simulink model "x64_32s_001_4ks"  (Simulink 7.4)
settings: Solver=ode45  StartTime=0.0  StopTime=100000  RelTol=1e-3
undefined symbols (not defined in the file): gcbh, ROACH, x64_32s_001_4ks_beam_mux_order_in_user_data_out, x64_32s_001_4ks_beam_mux_order_out_user_data_out, x64_32s_001_4ks_beam_mux_order_we_user_data_out, x64_32s_001_4ks_first_chan_user_data_out, x64_32s_001_4ks_gbe_dest_ip_user_data_out, x64_32s_001_4ks_gbe_dest_port_user_data_out, x64_32s_001_4ks_gbe_packet_cnt1_user_data_in, x64_32s_001_4ks_gbe_packet_cnt_user_data_in, x64_32s_001_4ks_heap_size_user_data_out, x64_32s_001_4ks_inst_seng_id0_user_data_in, x64_32s_001_4ks_last_chan_user_data_out, x64_32s_001_4ks_n_beams_user_data_out, x64_32s_001_4ks_qdr_in_cnt_user_data_in, x64_32s_001_4ks_status_user_data_in, x64_32s_001_4ks_x_window_mult_0_coeff_user_data_out, x64_32s_001_4ks_x_window_mult_1_coeff_user_data_out, x64_32s_001_4ks_x_window_mult_2_coeff_user_data_out, x64_32s_001_4ks_x_window_mult_3_coeff_user_data_out, x64_32s_001_4ks_xaui_cnt0_user_data_in, x64_32s_001_4ks_xaui_error_cnt0_user_data_in, x64_32s_001_4ks_xauibuf_out_cnt_user_data_in, x64_32s_001_4ks_xauibuf_window_cnt_user_data_in, x64_32s_001_4ks_y_window_mult_0_coeff_user_data_out, x64_32s_001_4ks_y_window_mult_1_coeff_user_data_out, x64_32s_001_4ks_y_window_mult_2_coeff_user_data_out, x64_32s_001_4ks_y_window_mult_3_coeff_user_data_out, x64_32s_001_4ks_y_window_mult_4_coeff_user_data_out, x64_32s_001_4ks_y_window_mult_5_coeff_user_data_out, x64_32s_001_4ks_y_window_mult_6_coeff_user_data_out, x64_32s_001_4ks_y_window_mult_7_coeff_user_data_out, xlBlockLoadCallback
libraries: xbsIndex_r4 x8559, casper_library_misc x308, casper_library_delays x33, casper_library_reorder x16, xps_library x5, ox_lib x1
(part 14 of 100)

== system x64_32s_001_4ks/beam_tvg/pow_sim_mux10 ==
  sync : Inport  OutDataType=fixdt(1, 16)  OutScaling=2^0
  din : Inport  Port=2  OutDataType=fixdt(1, 16)  OutScaling=2^0
  tvg_en : Inport  Port=3  OutDataType=fixdt(1, 16)  OutScaling=2^0
  new_window : Inport  Port=4  OutDataType=fixdt(1, 16)  OutScaling=2^0
  Convert : xbsIndex_r4/Convert  arith_type=Unsigned  n_bits=8  bin_pt=0  quantization=Truncate  overflow=Wrap  latency=0  dbl_ovrd=off  pipeline=off  block_type=convert
      settings: block_version=11.4
  Mux : xbsIndex_r4/Mux  inputs=2  en=off  latency=1  precision=Full  arith_type=Unsigned  n_bits=16  bin_pt=14  quantization=Truncate  overflow=Wrap  dbl_ovrd=off  block_type=mux
      settings: block_version=11.4
  beam_ctr : xbsIndex_r4/Counter  cnt_type=Free Running  cnt_to=Inf  operation=Up  start_count=0  cnt_by_val=1  arith_type=Unsigned  n_bits=ant_bits  bin_pt=0  load_pin=off  rst=on  en=off  explicit_period=on  period=1  dbl_ovrd=off  use_behavioral_HDL=off  implementation=Fabric  block_type=counter
      settings: block_version=11.4
  chan_ctr : xbsIndex_r4/Counter  cnt_type=Free Running  cnt_to=Inf  operation=Up  start_count=0  cnt_by_val=1  arith_type=Unsigned  n_bits=16  bin_pt=0  load_pin=off  rst=on  en=on  explicit_period=on  period=1  dbl_ovrd=off  use_behavioral_HDL=off  implementation=Fabric  block_type=counter
      settings: block_version=11.4
  imag : xbsIndex_r4/Concat  num_inputs=2  dbl_ovrd=off  block_type=concat
  real : xbsIndex_r4/Concat  num_inputs=3  dbl_ovrd=off  block_type=concat
  tv : xbsIndex_r4/Concat  num_inputs=2  dbl_ovrd=off  block_type=concat
  y_beam_val : xbsIndex_r4/Constant  arith_type=Unsigned  const=y_beam_val  n_bits=8  bin_pt=0  explicit_period=on  period=1  equ=P=C  opselect=C  inp2=PCIN>>17  opr=+  inp1=P  carry=CIN  dbl_ovrd=off  block_type=constant
      settings: block_version=11.4
  zero_pad : xbsIndex_r4/Constant  arith_type=Unsigned  const=0  n_bits=2  bin_pt=0  explicit_period=on  period=1  equ=P=C  opselect=C  inp2=PCIN>>17  opr=+  inp1=P  carry=CIN  dbl_ovrd=off  block_type=constant
      settings: block_version=11.4
  zero_pad1 : xbsIndex_r4/Constant  arith_type=Unsigned  const=0  n_bits=2  bin_pt=0  explicit_period=on  period=1  equ=P=C  opselect=C  inp2=PCIN>>17  opr=+  inp1=P  carry=CIN  dbl_ovrd=off  block_type=constant
      settings: block_version=11.4
  dout : Outport
  wires:
  imag/1 -> tv/2
  zero_pad1/1 -> imag/2
  tv/1 -> Mux/3
  zero_pad/1 -> real/3
  Convert/1 -> real/2
  y_beam_val/1 -> real/1
  din/1 -> Mux/2
  sync/1 -> chan_ctr/1
  beam_ctr/1 -> Convert/1
  tvg_en/1 -> Mux/1
  Mux/1 -> dout/1
  chan_ctr/1 -> imag/1
  real/1 -> tv/1
  new_window/1 -> chan_ctr/2, beam_ctr/1

== system x64_32s_001_4ks/beam_tvg/pow_sim_mux11 ==
  sync : Inport  OutDataType=fixdt(1, 16)  OutScaling=2^0
  din : Inport  Port=2  OutDataType=fixdt(1, 16)  OutScaling=2^0
  tvg_en : Inport  Port=3  OutDataType=fixdt(1, 16)  OutScaling=2^0
  new_window : Inport  Port=4  OutDataType=fixdt(1, 16)  OutScaling=2^0
  Convert : xbsIndex_r4/Convert  arith_type=Unsigned  n_bits=8  bin_pt=0  quantization=Truncate  overflow=Wrap  latency=0  dbl_ovrd=off  pipeline=off  block_type=convert
      settings: block_version=11.4
  Mux : xbsIndex_r4/Mux  inputs=2  en=off  latency=1  precision=Full  arith_type=Unsigned  n_bits=16  bin_pt=14  quantization=Truncate  overflow=Wrap  dbl_ovrd=off  block_type=mux
      settings: block_version=11.4
  beam_ctr : xbsIndex_r4/Counter  cnt_type=Free Running  cnt_to=Inf  operation=Up  start_count=0  cnt_by_val=1  arith_type=Unsigned  n_bits=ant_bits  bin_pt=0  load_pin=off  rst=on  en=off  explicit_period=on  period=1  dbl_ovrd=off  use_behavioral_HDL=off  implementation=Fabric  block_type=counter
      settings: block_version=11.4
  chan_ctr : xbsIndex_r4/Counter  cnt_type=Free Running  cnt_to=Inf  operation=Up  start_count=0  cnt_by_val=1  arith_type=Unsigned  n_bits=16  bin_pt=0  load_pin=off  rst=on  en=on  explicit_period=on  period=1  dbl_ovrd=off  use_behavioral_HDL=off  implementation=Fabric  block_type=counter
      settings: block_version=11.4
  imag : xbsIndex_r4/Concat  num_inputs=2  dbl_ovrd=off  block_type=concat
  real : xbsIndex_r4/Concat  num_inputs=3  dbl_ovrd=off  block_type=concat
  tv : xbsIndex_r4/Concat  num_inputs=2  dbl_ovrd=off  block_type=concat
  y_beam_val : xbsIndex_r4/Constant  arith_type=Unsigned  const=y_beam_val  n_bits=8  bin_pt=0  explicit_period=on  period=1  equ=P=C  opselect=C  inp2=PCIN>>17  opr=+  inp1=P  carry=CIN  dbl_ovrd=off  block_type=constant
      settings: block_version=11.4
  zero_pad : xbsIndex_r4/Constant  arith_type=Unsigned  const=0  n_bits=2  bin_pt=0  explicit_period=on  period=1  equ=P=C  opselect=C  inp2=PCIN>>17  opr=+  inp1=P  carry=CIN  dbl_ovrd=off  block_type=constant
      settings: block_version=11.4
  zero_pad1 : xbsIndex_r4/Constant  arith_type=Unsigned  const=0  n_bits=2  bin_pt=0  explicit_period=on  period=1  equ=P=C  opselect=C  inp2=PCIN>>17  opr=+  inp1=P  carry=CIN  dbl_ovrd=off  block_type=constant
      settings: block_version=11.4
  dout : Outport
  wires:
  imag/1 -> tv/2
  zero_pad1/1 -> imag/2
  tv/1 -> Mux/3
  zero_pad/1 -> real/3
  Convert/1 -> real/2
  y_beam_val/1 -> real/1
  din/1 -> Mux/2
  sync/1 -> chan_ctr/1
  beam_ctr/1 -> Convert/1
  tvg_en/1 -> Mux/1
  Mux/1 -> dout/1
  chan_ctr/1 -> imag/1
  real/1 -> tv/1
  new_window/1 -> chan_ctr/2, beam_ctr/1

== system x64_32s_001_4ks/beam_tvg/pow_sim_mux12 ==
  sync : Inport  OutDataType=fixdt(1, 16)  OutScaling=2^0
  din : Inport  Port=2  OutDataType=fixdt(1, 16)  OutScaling=2^0
  tvg_en : Inport  Port=3  OutDataType=fixdt(1, 16)  OutScaling=2^0
  new_window : Inport  Port=4  OutDataType=fixdt(1, 16)  OutScaling=2^0
  Convert : xbsIndex_r4/Convert  arith_type=Unsigned  n_bits=8  bin_pt=0  quantization=Truncate  overflow=Wrap  latency=0  dbl_ovrd=off  pipeline=off  block_type=convert
      settings: block_version=11.4
  Mux : xbsIndex_r4/Mux  inputs=2  en=off  latency=1  precision=Full  arith_type=Unsigned  n_bits=16  bin_pt=14  quantization=Truncate  overflow=Wrap  dbl_ovrd=off  block_type=mux
      settings: block_version=11.4
  beam_ctr : xbsIndex_r4/Counter  cnt_type=Free Running  cnt_to=Inf  operation=Up  start_count=0  cnt_by_val=1  arith_type=Unsigned  n_bits=ant_bits  bin_pt=0  load_pin=off  rst=on  en=off  explicit_period=on  period=1  dbl_ovrd=off  use_behavioral_HDL=off  implementation=Fabric  block_type=counter
      settings: block_version=11.4
  chan_ctr : xbsIndex_r4/Counter  cnt_type=Free Running  cnt_to=Inf  operation=Up  start_count=0  cnt_by_val=1  arith_type=Unsigned  n_bits=16  bin_pt=0  load_pin=off  rst=on  en=on  explicit_period=on  period=1  dbl_ovrd=off  use_behavioral_HDL=off  implementation=Fabric  block_type=counter
      settings: block_version=11.4
  imag : xbsIndex_r4/Concat  num_inputs=2  dbl_ovrd=off  block_type=concat
  real : xbsIndex_r4/Concat  num_inputs=3  dbl_ovrd=off  block_type=concat
  tv : xbsIndex_r4/Concat  num_inputs=2  dbl_ovrd=off  block_type=concat
  y_beam_val : xbsIndex_r4/Constant  arith_type=Unsigned  const=y_beam_val  n_bits=8  bin_pt=0  explicit_period=on  period=1  equ=P=C  opselect=C  inp2=PCIN>>17  opr=+  inp1=P  carry=CIN  dbl_ovrd=off  block_type=constant
      settings: block_version=11.4
  zero_pad : xbsIndex_r4/Constant  arith_type=Unsigned  const=0  n_bits=2  bin_pt=0  explicit_period=on  period=1  equ=P=C  opselect=C  inp2=PCIN>>17  opr=+  inp1=P  carry=CIN  dbl_ovrd=off  block_type=constant
      settings: block_version=11.4
  zero_pad1 : xbsIndex_r4/Constant  arith_type=Unsigned  const=0  n_bits=2  bin_pt=0  explicit_period=on  period=1  equ=P=C  opselect=C  inp2=PCIN>>17  opr=+  inp1=P  carry=CIN  dbl_ovrd=off  block_type=constant
      settings: block_version=11.4
  dout : Outport
  wires:
  new_window/1 -> beam_ctr/1, chan_ctr/2
  real/1 -> tv/1
  chan_ctr/1 -> imag/1
  Mux/1 -> dout/1
  tvg_en/1 -> Mux/1
  beam_ctr/1 -> Convert/1
  sync/1 -> chan_ctr/1
  din/1 -> Mux/2
  y_beam_val/1 -> real/1
  Convert/1 -> real/2
  zero_pad/1 -> real/3
  tv/1 -> Mux/3
  zero_pad1/1 -> imag/2
  imag/1 -> tv/2

== system x64_32s_001_4ks/beam_tvg/pow_sim_mux13 ==
  sync : Inport  OutDataType=fixdt(1, 16)  OutScaling=2^0
  din : Inport  Port=2  OutDataType=fixdt(1, 16)  OutScaling=2^0
  tvg_en : Inport  Port=3  OutDataType=fixdt(1, 16)  OutScaling=2^0
  new_window : Inport  Port=4  OutDataType=fixdt(1, 16)  OutScaling=2^0
  Convert : xbsIndex_r4/Convert  arith_type=Unsigned  n_bits=8  bin_pt=0  quantization=Truncate  overflow=Wrap  latency=0  dbl_ovrd=off  pipeline=off  block_type=convert
      settings: block_version=11.4
  Mux : xbsIndex_r4/Mux  inputs=2  en=off  latency=1  precision=Full  arith_type=Unsigned  n_bits=16  bin_pt=14  quantization=Truncate  overflow=Wrap  dbl_ovrd=off  block_type=mux
      settings: block_version=11.4
  beam_ctr : xbsIndex_r4/Counter  cnt_type=Free Running  cnt_to=Inf  operation=Up  start_count=0  cnt_by_val=1  arith_type=Unsigned  n_bits=ant_bits  bin_pt=0  load_pin=off  rst=on  en=off  explicit_period=on  period=1  dbl_ovrd=off  use_behavioral_HDL=off  implementation=Fabric  block_type=counter
      settings: block_version=11.4
  chan_ctr : xbsIndex_r4/Counter  cnt_type=Free Running  cnt_to=Inf  operation=Up  start_count=0  cnt_by_val=1  arith_type=Unsigned  n_bits=16  bin_pt=0  load_pin=off  rst=on  en=on  explicit_period=on  period=1  dbl_ovrd=off  use_behavioral_HDL=off  implementation=Fabric  block_type=counter
      settings: block_version=11.4
  imag : xbsIndex_r4/Concat  num_inputs=2  dbl_ovrd=off  block_type=concat
  real : xbsIndex_r4/Concat  num_inputs=3  dbl_ovrd=off  block_type=concat
  tv : xbsIndex_r4/Concat  num_inputs=2  dbl_ovrd=off  block_type=concat
  y_beam_val : xbsIndex_r4/Constant  arith_type=Unsigned  const=y_beam_val  n_bits=8  bin_pt=0  explicit_period=on  period=1  equ=P=C  opselect=C  inp2=PCIN>>17  opr=+  inp1=P  carry=CIN  dbl_ovrd=off  block_type=constant
      settings: block_version=11.4
  zero_pad : xbsIndex_r4/Constant  arith_type=Unsigned  const=0  n_bits=2  bin_pt=0  explicit_period=on  period=1  equ=P=C  opselect=C  inp2=PCIN>>17  opr=+  inp1=P  carry=CIN  dbl_ovrd=off  block_type=constant
      settings: block_version=11.4
  zero_pad1 : xbsIndex_r4/Constant  arith_type=Unsigned  const=0  n_bits=2  bin_pt=0  explicit_period=on  period=1  equ=P=C  opselect=C  inp2=PCIN>>17  opr=+  inp1=P  carry=CIN  dbl_ovrd=off  block_type=constant
      settings: block_version=11.4
  dout : Outport
  wires:
  new_window/1 -> beam_ctr/1, chan_ctr/2
  real/1 -> tv/1
  chan_ctr/1 -> imag/1
  Mux/1 -> dout/1
  tvg_en/1 -> Mux/1
  beam_ctr/1 -> Convert/1
  sync/1 -> chan_ctr/1
  din/1 -> Mux/2
  y_beam_val/1 -> real/1
  Convert/1 -> real/2
  zero_pad/1 -> real/3
  tv/1 -> Mux/3
  zero_pad1/1 -> imag/2
  imag/1 -> tv/2

== system x64_32s_001_4ks/beam_tvg/pow_sim_mux14 ==
  sync : Inport  OutDataType=fixdt(1, 16)  OutScaling=2^0
  din : Inport  Port=2  OutDataType=fixdt(1, 16)  OutScaling=2^0
  tvg_en : Inport  Port=3  OutDataType=fixdt(1, 16)  OutScaling=2^0
  new_window : Inport  Port=4  OutDataType=fixdt(1, 16)  OutScaling=2^0
  Convert : xbsIndex_r4/Convert  arith_type=Unsigned  n_bits=8  bin_pt=0  quantization=Truncate  overflow=Wrap  latency=0  dbl_ovrd=off  pipeline=off  block_type=convert
      settings: block_version=11.4
  Mux : xbsIndex_r4/Mux  inputs=2  en=off  latency=1  precision=Full  arith_type=Unsigned  n_bits=16  bin_pt=14  quantization=Truncate  overflow=Wrap  dbl_ovrd=off  block_type=mux
      settings: block_version=11.4
  beam_ctr : xbsIndex_r4/Counter  cnt_type=Free Running  cnt_to=Inf  operation=Up  start_count=0  cnt_by_val=1  arith_type=Unsigned  n_bits=ant_bits  bin_pt=0  load_pin=off  rst=on  en=off  explicit_period=on  period=1  dbl_ovrd=off  use_behavioral_HDL=off  implementation=Fabric  block_type=counter
      settings: block_version=11.4
  chan_ctr : xbsIndex_r4/Counter  cnt_type=Free Running  cnt_to=Inf  operation=Up  start_count=0  cnt_by_val=1  arith_type=Unsigned  n_bits=16  bin_pt=0  load_pin=off  rst=on  en=on  explicit_period=on  period=1  dbl_ovrd=off  use_behavioral_HDL=off  implementation=Fabric  block_type=counter
      settings: block_version=11.4
  imag : xbsIndex_r4/Concat  num_inputs=2  dbl_ovrd=off  block_type=concat
  real : xbsIndex_r4/Concat  num_inputs=3  dbl_ovrd=off  block_type=concat
  tv : xbsIndex_r4/Concat  num_inputs=2  dbl_ovrd=off  block_type=concat
  y_beam_val : xbsIndex_r4/Constant  arith_type=Unsigned  const=y_beam_val  n_bits=8  bin_pt=0  explicit_period=on  period=1  equ=P=C  opselect=C  inp2=PCIN>>17  opr=+  inp1=P  carry=CIN  dbl_ovrd=off  block_type=constant
      settings: block_version=11.4
  zero_pad : xbsIndex_r4/Constant  arith_type=Unsigned  const=0  n_bits=2  bin_pt=0  explicit_period=on  period=1  equ=P=C  opselect=C  inp2=PCIN>>17  opr=+  inp1=P  carry=CIN  dbl_ovrd=off  block_type=constant
      settings: block_version=11.4
  zero_pad1 : xbsIndex_r4/Constant  arith_type=Unsigned  const=0  n_bits=2  bin_pt=0  explicit_period=on  period=1  equ=P=C  opselect=C  inp2=PCIN>>17  opr=+  inp1=P  carry=CIN  dbl_ovrd=off  block_type=constant
      settings: block_version=11.4
  dout : Outport
  wires:
  new_window/1 -> beam_ctr/1, chan_ctr/2
  real/1 -> tv/1
  chan_ctr/1 -> imag/1
  Mux/1 -> dout/1
  tvg_en/1 -> Mux/1
  beam_ctr/1 -> Convert/1
  sync/1 -> chan_ctr/1
  din/1 -> Mux/2
  y_beam_val/1 -> real/1
  Convert/1 -> real/2
  zero_pad/1 -> real/3
  tv/1 -> Mux/3
  zero_pad1/1 -> imag/2
  imag/1 -> tv/2

== system x64_32s_001_4ks/beam_tvg/pow_sim_mux15 ==
  sync : Inport  OutDataType=fixdt(1, 16)  OutScaling=2^0
  din : Inport  Port=2  OutDataType=fixdt(1, 16)  OutScaling=2^0
  tvg_en : Inport  Port=3  OutDataType=fixdt(1, 16)  OutScaling=2^0
  new_window : Inport  Port=4  OutDataType=fixdt(1, 16)  OutScaling=2^0
  Convert : xbsIndex_r4/Convert  arith_type=Unsigned  n_bits=8  bin_pt=0  quantization=Truncate  overflow=Wrap  latency=0  dbl_ovrd=off  pipeline=off  block_type=convert
      settings: block_version=11.4
  Mux : xbsIndex_r4/Mux  inputs=2  en=off  latency=1  precision=Full  arith_type=Unsigned  n_bits=16  bin_pt=14  quantization=Truncate  overflow=Wrap  dbl_ovrd=off  block_type=mux
      settings: block_version=11.4
  beam_ctr : xbsIndex_r4/Counter  cnt_type=Free Running  cnt_to=Inf  operation=Up  start_count=0  cnt_by_val=1  arith_type=Unsigned  n_bits=ant_bits  bin_pt=0  load_pin=off  rst=on  en=off  explicit_period=on  period=1  dbl_ovrd=off  use_behavioral_HDL=off  implementation=Fabric  block_type=counter
      settings: block_version=11.4
  chan_ctr : xbsIndex_r4/Counter  cnt_type=Free Running  cnt_to=Inf  operation=Up  start_count=0  cnt_by_val=1  arith_type=Unsigned  n_bits=16  bin_pt=0  load_pin=off  rst=on  en=on  explicit_period=on  period=1  dbl_ovrd=off  use_behavioral_HDL=off  implementation=Fabric  block_type=counter
      settings: block_version=11.4
  imag : xbsIndex_r4/Concat  num_inputs=2  dbl_ovrd=off  block_type=concat
  real : xbsIndex_r4/Concat  num_inputs=3  dbl_ovrd=off  block_type=concat
  tv : xbsIndex_r4/Concat  num_inputs=2  dbl_ovrd=off  block_type=concat
  y_beam_val : xbsIndex_r4/Constant  arith_type=Unsigned  const=y_beam_val  n_bits=8  bin_pt=0  explicit_period=on  period=1  equ=P=C  opselect=C  inp2=PCIN>>17  opr=+  inp1=P  carry=CIN  dbl_ovrd=off  block_type=constant
      settings: block_version=11.4
  zero_pad : xbsIndex_r4/Constant  arith_type=Unsigned  const=0  n_bits=2  bin_pt=0  explicit_period=on  period=1  equ=P=C  opselect=C  inp2=PCIN>>17  opr=+  inp1=P  carry=CIN  dbl_ovrd=off  block_type=constant
      settings: block_version=11.4
  zero_pad1 : xbsIndex_r4/Constant  arith_type=Unsigned  const=0  n_bits=2  bin_pt=0  explicit_period=on  period=1  equ=P=C  opselect=C  inp2=PCIN>>17  opr=+  inp1=P  carry=CIN  dbl_ovrd=off  block_type=constant
      settings: block_version=11.4
  dout : Outport
  wires:
  imag/1 -> tv/2
  zero_pad1/1 -> imag/2
  tv/1 -> Mux/3
  zero_pad/1 -> real/3
  Convert/1 -> real/2
  y_beam_val/1 -> real/1
  din/1 -> Mux/2
  sync/1 -> chan_ctr/1
  beam_ctr/1 -> Convert/1
  tvg_en/1 -> Mux/1
  Mux/1 -> dout/1
  chan_ctr/1 -> imag/1
  real/1 -> tv/1
  new_window/1 -> chan_ctr/2, beam_ctr/1

== system x64_32s_001_4ks/beam_tvg/pow_sim_mux16 ==
  sync : Inport  OutDataType=fixdt(1, 16)  OutScaling=2^0
  din : Inport  Port=2  OutDataType=fixdt(1, 16)  OutScaling=2^0
  tvg_en : Inport  Port=3  OutDataType=fixdt(1, 16)  OutScaling=2^0
  new_window : Inport  Port=4  OutDataType=fixdt(1, 16)  OutScaling=2^0
  Convert : xbsIndex_r4/Convert  arith_type=Unsigned  n_bits=8  bin_pt=0  quantization=Truncate  overflow=Wrap  latency=0  dbl_ovrd=off  pipeline=off  block_type=convert
      settings: block_version=11.4
  Mux : xbsIndex_r4/Mux  inputs=2  en=off  latency=1  precision=Full  arith_type=Unsigned  n_bits=16  bin_pt=14  quantization=Truncate  overflow=Wrap  dbl_ovrd=off  block_type=mux
      settings: block_version=11.4
  beam_ctr : xbsIndex_r4/Counter  cnt_type=Free Running  cnt_to=Inf  operation=Up  start_count=0  cnt_by_val=1  arith_type=Unsigned  n_bits=ant_bits  bin_pt=0  load_pin=off  rst=on  en=off  explicit_period=on  period=1  dbl_ovrd=off  use_behavioral_HDL=off  implementation=Fabric  block_type=counter
      settings: block_version=11.4
  chan_ctr : xbsIndex_r4/Counter  cnt_type=Free Running  cnt_to=Inf  operation=Up  start_count=0  cnt_by_val=1  arith_type=Unsigned  n_bits=16  bin_pt=0  load_pin=off  rst=on  en=on  explicit_period=on  period=1  dbl_ovrd=off  use_behavioral_HDL=off  implementation=Fabric  block_type=counter
      settings: block_version=11.4
  imag : xbsIndex_r4/Concat  num_inputs=2  dbl_ovrd=off  block_type=concat
  real : xbsIndex_r4/Concat  num_inputs=3  dbl_ovrd=off  block_type=concat
  tv : xbsIndex_r4/Concat  num_inputs=2  dbl_ovrd=off  block_type=concat
  y_beam_val : xbsIndex_r4/Constant  arith_type=Unsigned  const=y_beam_val  n_bits=8  bin_pt=0  explicit_period=on  period=1  equ=P=C  opselect=C  inp2=PCIN>>17  opr=+  inp1=P  carry=CIN  dbl_ovrd=off  block_type=constant
      settings: block_version=11.4
  zero_pad : xbsIndex_r4/Constant  arith_type=Unsigned  const=0  n_bits=2  bin_pt=0  explicit_period=on  period=1  equ=P=C  opselect=C  inp2=PCIN>>17  opr=+  inp1=P  carry=CIN  dbl_ovrd=off  block_type=constant
      settings: block_version=11.4
  zero_pad1 : xbsIndex_r4/Constant  arith_type=Unsigned  const=0  n_bits=2  bin_pt=0  explicit_period=on  period=1  equ=P=C  opselect=C  inp2=PCIN>>17  opr=+  inp1=P  carry=CIN  dbl_ovrd=off  block_type=constant
      settings: block_version=11.4
  dout : Outport
  wires:
  imag/1 -> tv/2
  zero_pad1/1 -> imag/2
  tv/1 -> Mux/3
  zero_pad/1 -> real/3
  Convert/1 -> real/2
  y_beam_val/1 -> real/1
  din/1 -> Mux/2
  sync/1 -> chan_ctr/1
  beam_ctr/1 -> Convert/1
  tvg_en/1 -> Mux/1
  Mux/1 -> dout/1
  chan_ctr/1 -> imag/1
  real/1 -> tv/1
  new_window/1 -> chan_ctr/2, beam_ctr/1

== system x64_32s_001_4ks/beam_tvg/pow_sim_mux2 ==
  sync : Inport  OutDataType=fixdt(1, 16)  OutScaling=2^0
  din : Inport  Port=2  OutDataType=fixdt(1, 16)  OutScaling=2^0
  tvg_en : Inport  Port=3  OutDataType=fixdt(1, 16)  OutScaling=2^0
  new_window : Inport  Port=4  OutDataType=fixdt(1, 16)  OutScaling=2^0
  Convert : xbsIndex_r4/Convert  arith_type=Unsigned  n_bits=8  bin_pt=0  quantization=Truncate  overflow=Wrap  latency=0  dbl_ovrd=off  pipeline=off  block_type=convert
      settings: block_version=11.4
  Mux : xbsIndex_r4/Mux  inputs=2  en=off  latency=1  precision=Full  arith_type=Unsigned  n_bits=16  bin_pt=14  quantization=Truncate  overflow=Wrap  dbl_ovrd=off  block_type=mux
      settings: block_version=11.4
  beam_ctr : xbsIndex_r4/Counter  cnt_type=Free Running  cnt_to=Inf  operation=Up  start_count=0  cnt_by_val=1  arith_type=Unsigned  n_bits=ant_bits  bin_pt=0  load_pin=off  rst=on  en=off  explicit_period=on  period=1  dbl_ovrd=off  use_behavioral_HDL=off  implementation=Fabric  block_type=counter
      settings: block_version=11.4
  chan_ctr : xbsIndex_r4/Counter  cnt_type=Free Running  cnt_to=Inf  operation=Up  start_count=0  cnt_by_val=1  arith_type=Unsigned  n_bits=16  bin_pt=0  load_pin=off  rst=on  en=on  explicit_period=on  period=1  dbl_ovrd=off  use_behavioral_HDL=off  implementation=Fabric  block_type=counter
      settings: block_version=11.4
  imag : xbsIndex_r4/Concat  num_inputs=2  dbl_ovrd=off  block_type=concat
  real : xbsIndex_r4/Concat  num_inputs=3  dbl_ovrd=off  block_type=concat
  tv : xbsIndex_r4/Concat  num_inputs=2  dbl_ovrd=off  block_type=concat
  y_beam_val : xbsIndex_r4/Constant  arith_type=Unsigned  const=y_beam_val  n_bits=8  bin_pt=0  explicit_period=on  period=1  equ=P=C  opselect=C  inp2=PCIN>>17  opr=+  inp1=P  carry=CIN  dbl_ovrd=off  block_type=constant
      settings: block_version=11.4
  zero_pad : xbsIndex_r4/Constant  arith_type=Unsigned  const=0  n_bits=2  bin_pt=0  explicit_period=on  period=1  equ=P=C  opselect=C  inp2=PCIN>>17  opr=+  inp1=P  carry=CIN  dbl_ovrd=off  block_type=constant
      settings: block_version=11.4
  zero_pad1 : xbsIndex_r4/Constant  arith_type=Unsigned  const=0  n_bits=2  bin_pt=0  explicit_period=on  period=1  equ=P=C  opselect=C  inp2=PCIN>>17  opr=+  inp1=P  carry=CIN  dbl_ovrd=off  block_type=constant
      settings: block_version=11.4
  dout : Outport
  wires:
  imag/1 -> tv/2
  zero_pad1/1 -> imag/2
  tv/1 -> Mux/3
  zero_pad/1 -> real/3
  Convert/1 -> real/2
  y_beam_val/1 -> real/1
  din/1 -> Mux/2
  sync/1 -> chan_ctr/1
  beam_ctr/1 -> Convert/1
  tvg_en/1 -> Mux/1
  Mux/1 -> dout/1
  chan_ctr/1 -> imag/1
  real/1 -> tv/1
  new_window/1 -> chan_ctr/2, beam_ctr/1

== system x64_32s_001_4ks/beam_tvg/pow_sim_mux3 ==
  sync : Inport  OutDataType=fixdt(1, 16)  OutScaling=2^0
  din : Inport  Port=2  OutDataType=fixdt(1, 16)  OutScaling=2^0
  tvg_en : Inport  Port=3  OutDataType=fixdt(1, 16)  OutScaling=2^0
  new_window : Inport  Port=4  OutDataType=fixdt(1, 16)  OutScaling=2^0
  Convert : xbsIndex_r4/Convert  arith_type=Unsigned  n_bits=8  bin_pt=0  quantization=Truncate  overflow=Wrap  latency=0  dbl_ovrd=off  pipeline=off  block_type=convert
      settings: block_version=11.4
  Mux : xbsIndex_r4/Mux  inputs=2  en=off  latency=1  precision=Full  arith_type=Unsigned  n_bits=16  bin_pt=14  quantization=Truncate  overflow=Wrap  dbl_ovrd=off  block_type=mux
      settings: block_version=11.4
  beam_ctr : xbsIndex_r4/Counter  cnt_type=Free Running  cnt_to=Inf  operation=Up  start_count=0  cnt_by_val=1  arith_type=Unsigned  n_bits=ant_bits  bin_pt=0  load_pin=off  rst=on  en=off  explicit_period=on  period=1  dbl_ovrd=off  use_behavioral_HDL=off  implementation=Fabric  block_type=counter
      settings: block_version=11.4
  chan_ctr : xbsIndex_r4/Counter  cnt_type=Free Running  cnt_to=Inf  operation=Up  start_count=0  cnt_by_val=1  arith_type=Unsigned  n_bits=16  bin_pt=0  load_pin=off  rst=on  en=on  explicit_period=on  period=1  dbl_ovrd=off  use_behavioral_HDL=off  implementation=Fabric  block_type=counter
      settings: block_version=11.4
  imag : xbsIndex_r4/Concat  num_inputs=2  dbl_ovrd=off  block_type=concat
  real : xbsIndex_r4/Concat  num_inputs=3  dbl_ovrd=off  block_type=concat
  tv : xbsIndex_r4/Concat  num_inputs=2  dbl_ovrd=off  block_type=concat
  y_beam_val : xbsIndex_r4/Constant  arith_type=Unsigned  const=y_beam_val  n_bits=8  bin_pt=0  explicit_period=on  period=1  equ=P=C  opselect=C  inp2=PCIN>>17  opr=+  inp1=P  carry=CIN  dbl_ovrd=off  block_type=constant
      settings: block_version=11.4
  zero_pad : xbsIndex_r4/Constant  arith_type=Unsigned  const=0  n_bits=2  bin_pt=0  explicit_period=on  period=1  equ=P=C  opselect=C  inp2=PCIN>>17  opr=+  inp1=P  carry=CIN  dbl_ovrd=off  block_type=constant
      settings: block_version=11.4
  zero_pad1 : xbsIndex_r4/Constant  arith_type=Unsigned  const=0  n_bits=2  bin_pt=0  explicit_period=on  period=1  equ=P=C  opselect=C  inp2=PCIN>>17  opr=+  inp1=P  carry=CIN  dbl_ovrd=off  block_type=constant
      settings: block_version=11.4
  dout : Outport
  wires:
  imag/1 -> tv/2
  zero_pad1/1 -> imag/2
  tv/1 -> Mux/3
  zero_pad/1 -> real/3
  Convert/1 -> real/2
  y_beam_val/1 -> real/1
  din/1 -> Mux/2
  sync/1 -> chan_ctr/1
  beam_ctr/1 -> Convert/1
  tvg_en/1 -> Mux/1
  Mux/1 -> dout/1
  chan_ctr/1 -> imag/1
  real/1 -> tv/1
  new_window/1 -> chan_ctr/2, beam_ctr/1

== system x64_32s_001_4ks/beam_tvg/pow_sim_mux4 ==
  sync : Inport  OutDataType=fixdt(1, 16)  OutScaling=2^0
  din : Inport  Port=2  OutDataType=fixdt(1, 16)  OutScaling=2^0
  tvg_en : Inport  Port=3  OutDataType=fixdt(1, 16)  OutScaling=2^0
  new_window : Inport  Port=4  OutDataType=fixdt(1, 16)  OutScaling=2^0
  Convert : xbsIndex_r4/Convert  arith_type=Unsigned  n_bits=8  bin_pt=0  quantization=Truncate  overflow=Wrap  latency=0  dbl_ovrd=off  pipeline=off  block_type=convert
      settings: block_version=11.4
  Mux : xbsIndex_r4/Mux  inputs=2  en=off  latency=1  precision=Full  arith_type=Unsigned  n_bits=16  bin_pt=14  quantization=Truncate  overflow=Wrap  dbl_ovrd=off  block_type=mux
      settings: block_version=11.4
  beam_ctr : xbsIndex_r4/Counter  cnt_type=Free Running  cnt_to=Inf  operation=Up  start_count=0  cnt_by_val=1  arith_type=Unsigned  n_bits=ant_bits  bin_pt=0  load_pin=off  rst=on  en=off  explicit_period=on  period=1  dbl_ovrd=off  use_behavioral_HDL=off  implementation=Fabric  block_type=counter
      settings: block_version=11.4
  chan_ctr : xbsIndex_r4/Counter  cnt_type=Free Running  cnt_to=Inf  operation=Up  start_count=0  cnt_by_val=1  arith_type=Unsigned  n_bits=16  bin_pt=0  load_pin=off  rst=on  en=on  explicit_period=on  period=1  dbl_ovrd=off  use_behavioral_HDL=off  implementation=Fabric  block_type=counter
      settings: block_version=11.4
  imag : xbsIndex_r4/Concat  num_inputs=2  dbl_ovrd=off  block_type=concat
  real : xbsIndex_r4/Concat  num_inputs=3  dbl_ovrd=off  block_type=concat
  tv : xbsIndex_r4/Concat  num_inputs=2  dbl_ovrd=off  block_type=concat
  y_beam_val : xbsIndex_r4/Constant  arith_type=Unsigned  const=y_beam_val  n_bits=8  bin_pt=0  explicit_period=on  period=1  equ=P=C  opselect=C  inp2=PCIN>>17  opr=+  inp1=P  carry=CIN  dbl_ovrd=off  block_type=constant
      settings: block_version=11.4
  zero_pad : xbsIndex_r4/Constant  arith_type=Unsigned  const=0  n_bits=2  bin_pt=0  explicit_period=on  period=1  equ=P=C  opselect=C  inp2=PCIN>>17  opr=+  inp1=P  carry=CIN  dbl_ovrd=off  block_type=constant
      settings: block_version=11.4
  zero_pad1 : xbsIndex_r4/Constant  arith_type=Unsigned  const=0  n_bits=2  bin_pt=0  explicit_period=on  period=1  equ=P=C  opselect=C  inp2=PCIN>>17  opr=+  inp1=P  carry=CIN  dbl_ovrd=off  block_type=constant
      settings: block_version=11.4
  dout : Outport
  wires:
  new_window/1 -> beam_ctr/1, chan_ctr/2
  real/1 -> tv/1
  chan_ctr/1 -> imag/1
  Mux/1 -> dout/1
  tvg_en/1 -> Mux/1
  beam_ctr/1 -> Convert/1
  sync/1 -> chan_ctr/1
  din/1 -> Mux/2
  y_beam_val/1 -> real/1
  Convert/1 -> real/2
  zero_pad/1 -> real/3
  tv/1 -> Mux/3
  zero_pad1/1 -> imag/2
  imag/1 -> tv/2

== system x64_32s_001_4ks/beam_tvg/pow_sim_mux5 ==
  sync : Inport  OutDataType=fixdt(1, 16)  OutScaling=2^0
  din : Inport  Port=2  OutDataType=fixdt(1, 16)  OutScaling=2^0
  tvg_en : Inport  Port=3  OutDataType=fixdt(1, 16)  OutScaling=2^0
  new_window : Inport  Port=4  OutDataType=fixdt(1, 16)  OutScaling=2^0
  Convert : xbsIndex_r4/Convert  arith_type=Unsigned  n_bits=8  bin_pt=0  quantization=Truncate  overflow=Wrap  latency=0  dbl_ovrd=off  pipeline=off  block_type=convert
      settings: block_version=11.4
  Mux : xbsIndex_r4/Mux  inputs=2  en=off  latency=1  precision=Full  arith_type=Unsigned  n_bits=16  bin_pt=14  quantization=Truncate  overflow=Wrap  dbl_ovrd=off  block_type=mux
      settings: block_version=11.4
  beam_ctr : xbsIndex_r4/Counter  cnt_type=Free Running  cnt_to=Inf  operation=Up  start_count=0  cnt_by_val=1  arith_type=Unsigned  n_bits=ant_bits  bin_pt=0  load_pin=off  rst=on  en=off  explicit_period=on  period=1  dbl_ovrd=off  use_behavioral_HDL=off  implementation=Fabric  block_type=counter
      settings: block_version=11.4
  chan_ctr : xbsIndex_r4/Counter  cnt_type=Free Running  cnt_to=Inf  operation=Up  start_count=0  cnt_by_val=1  arith_type=Unsigned  n_bits=16  bin_pt=0  load_pin=off  rst=on  en=on  explicit_period=on  period=1  dbl_ovrd=off  use_behavioral_HDL=off  implementation=Fabric  block_type=counter
      settings: block_version=11.4
  imag : xbsIndex_r4/Concat  num_inputs=2  dbl_ovrd=off  block_type=concat
  real : xbsIndex_r4/Concat  num_inputs=3  dbl_ovrd=off  block_type=concat
  tv : xbsIndex_r4/Concat  num_inputs=2  dbl_ovrd=off  block_type=concat
  y_beam_val : xbsIndex_r4/Constant  arith_type=Unsigned  const=y_beam_val  n_bits=8  bin_pt=0  explicit_period=on  period=1  equ=P=C  opselect=C  inp2=PCIN>>17  opr=+  inp1=P  carry=CIN  dbl_ovrd=off  block_type=constant
      settings: block_version=11.4
  zero_pad : xbsIndex_r4/Constant  arith_type=Unsigned  const=0  n_bits=2  bin_pt=0  explicit_period=on  period=1  equ=P=C  opselect=C  inp2=PCIN>>17  opr=+  inp1=P  carry=CIN  dbl_ovrd=off  block_type=constant
      settings: block_version=11.4
  zero_pad1 : xbsIndex_r4/Constant  arith_type=Unsigned  const=0  n_bits=2  bin_pt=0  explicit_period=on  period=1  equ=P=C  opselect=C  inp2=PCIN>>17  opr=+  inp1=P  carry=CIN  dbl_ovrd=off  block_type=constant
      settings: block_version=11.4
  dout : Outport
  wires:
  imag/1 -> tv/2
  zero_pad1/1 -> imag/2
  tv/1 -> Mux/3
  zero_pad/1 -> real/3
  Convert/1 -> real/2
  y_beam_val/1 -> real/1
  din/1 -> Mux/2
  sync/1 -> chan_ctr/1
  beam_ctr/1 -> Convert/1
  tvg_en/1 -> Mux/1
  Mux/1 -> dout/1
  chan_ctr/1 -> imag/1
  real/1 -> tv/1
  new_window/1 -> chan_ctr/2, beam_ctr/1

== system x64_32s_001_4ks/beam_tvg/pow_sim_mux6 ==
  sync : Inport  OutDataType=fixdt(1, 16)  OutScaling=2^0
  din : Inport  Port=2  OutDataType=fixdt(1, 16)  OutScaling=2^0
  tvg_en : Inport  Port=3  OutDataType=fixdt(1, 16)  OutScaling=2^0
  new_window : Inport  Port=4  OutDataType=fixdt(1, 16)  OutScaling=2^0
  Convert : xbsIndex_r4/Convert  arith_type=Unsigned  n_bits=8  bin_pt=0  quantization=Truncate  overflow=Wrap  latency=0  dbl_ovrd=off  pipeline=off  block_type=convert
      settings: block_version=11.4
  Mux : xbsIndex_r4/Mux  inputs=2  en=off  latency=1  precision=Full  arith_type=Unsigned  n_bits=16  bin_pt=14  quantization=Truncate  overflow=Wrap  dbl_ovrd=off  block_type=mux
      settings: block_version=11.4
  beam_ctr : xbsIndex_r4/Counter  cnt_type=Free Running  cnt_to=Inf  operation=Up  start_count=0  cnt_by_val=1  arith_type=Unsigned  n_bits=ant_bits  bin_pt=0  load_pin=off  rst=on  en=off  explicit_period=on  period=1  dbl_ovrd=off  use_behavioral_HDL=off  implementation=Fabric  block_type=counter
      settings: block_version=11.4
  chan_ctr : xbsIndex_r4/Counter  cnt_type=Free Running  cnt_to=Inf  operation=Up  start_count=0  cnt_by_val=1  arith_type=Unsigned  n_bits=16  bin_pt=0  load_pin=off  rst=on  en=on  explicit_period=on  period=1  dbl_ovrd=off  use_behavioral_HDL=off  implementation=Fabric  block_type=counter
      settings: block_version=11.4
  imag : xbsIndex_r4/Concat  num_inputs=2  dbl_ovrd=off  block_type=concat
  real : xbsIndex_r4/Concat  num_inputs=3  dbl_ovrd=off  block_type=concat
  tv : xbsIndex_r4/Concat  num_inputs=2  dbl_ovrd=off  block_type=concat
  y_beam_val : xbsIndex_r4/Constant  arith_type=Unsigned  const=y_beam_val  n_bits=8  bin_pt=0  explicit_period=on  period=1  equ=P=C  opselect=C  inp2=PCIN>>17  opr=+  inp1=P  carry=CIN  dbl_ovrd=off  block_type=constant
      settings: block_version=11.4
  zero_pad : xbsIndex_r4/Constant  arith_type=Unsigned  const=0  n_bits=2  bin_pt=0  explicit_period=on  period=1  equ=P=C  opselect=C  inp2=PCIN>>17  opr=+  inp1=P  carry=CIN  dbl_ovrd=off  block_type=constant
      settings: block_version=11.4
  zero_pad1 : xbsIndex_r4/Constant  arith_type=Unsigned  const=0  n_bits=2  bin_pt=0  explicit_period=on  period=1  equ=P=C  opselect=C  inp2=PCIN>>17  opr=+  inp1=P  carry=CIN  dbl_ovrd=off  block_type=constant
      settings: block_version=11.4
  dout : Outport
  wires:
  new_window/1 -> beam_ctr/1, chan_ctr/2
  real/1 -> tv/1
  chan_ctr/1 -> imag/1
  Mux/1 -> dout/1
  tvg_en/1 -> Mux/1
  beam_ctr/1 -> Convert/1
  sync/1 -> chan_ctr/1
  din/1 -> Mux/2
  y_beam_val/1 -> real/1
  Convert/1 -> real/2
  zero_pad/1 -> real/3
  tv/1 -> Mux/3
  zero_pad1/1 -> imag/2
  imag/1 -> tv/2

== system x64_32s_001_4ks/beam_tvg/pow_sim_mux7 ==
  sync : Inport  OutDataType=fixdt(1, 16)  OutScaling=2^0
  din : Inport  Port=2  OutDataType=fixdt(1, 16)  OutScaling=2^0
  tvg_en : Inport  Port=3  OutDataType=fixdt(1, 16)  OutScaling=2^0
  new_window : Inport  Port=4  OutDataType=fixdt(1, 16)  OutScaling=2^0
  Convert : xbsIndex_r4/Convert  arith_type=Unsigned  n_bits=8  bin_pt=0  quantization=Truncate  overflow=Wrap  latency=0  dbl_ovrd=off  pipeline=off  block_type=convert
      settings: block_version=11.4
  Mux : xbsIndex_r4/Mux  inputs=2  en=off  latency=1  precision=Full  arith_type=Unsigned  n_bits=16  bin_pt=14  quantization=Truncate  overflow=Wrap  dbl_ovrd=off  block_type=mux
      settings: block_version=11.4
  beam_ctr : xbsIndex_r4/Counter  cnt_type=Free Running  cnt_to=Inf  operation=Up  start_count=0  cnt_by_val=1  arith_type=Unsigned  n_bits=ant_bits  bin_pt=0  load_pin=off  rst=on  en=off  explicit_period=on  period=1  dbl_ovrd=off  use_behavioral_HDL=off  implementation=Fabric  block_type=counter
      settings: block_version=11.4
  chan_ctr : xbsIndex_r4/Counter  cnt_type=Free Running  cnt_to=Inf  operation=Up  start_count=0  cnt_by_val=1  arith_type=Unsigned  n_bits=16  bin_pt=0  load_pin=off  rst=on  en=on  explicit_period=on  period=1  dbl_ovrd=off  use_behavioral_HDL=off  implementation=Fabric  block_type=counter
      settings: block_version=11.4
  imag : xbsIndex_r4/Concat  num_inputs=2  dbl_ovrd=off  block_type=concat
  real : xbsIndex_r4/Concat  num_inputs=3  dbl_ovrd=off  block_type=concat
  tv : xbsIndex_r4/Concat  num_inputs=2  dbl_ovrd=off  block_type=concat
  y_beam_val : xbsIndex_r4/Constant  arith_type=Unsigned  const=y_beam_val  n_bits=8  bin_pt=0  explicit_period=on  period=1  equ=P=C  opselect=C  inp2=PCIN>>17  opr=+  inp1=P  carry=CIN  dbl_ovrd=off  block_type=constant
      settings: block_version=11.4
  zero_pad : xbsIndex_r4/Constant  arith_type=Unsigned  const=0  n_bits=2  bin_pt=0  explicit_period=on  period=1  equ=P=C  opselect=C  inp2=PCIN>>17  opr=+  inp1=P  carry=CIN  dbl_ovrd=off  block_type=constant
      settings: block_version=11.4
  zero_pad1 : xbsIndex_r4/Constant  arith_type=Unsigned  const=0  n_bits=2  bin_pt=0  explicit_period=on  period=1  equ=P=C  opselect=C  inp2=PCIN>>17  opr=+  inp1=P  carry=CIN  dbl_ovrd=off  block_type=constant
      settings: block_version=11.4
  dout : Outport
  wires:
  new_window/1 -> beam_ctr/1, chan_ctr/2
  real/1 -> tv/1
  chan_ctr/1 -> imag/1
  Mux/1 -> dout/1
  tvg_en/1 -> Mux/1
  beam_ctr/1 -> Convert/1
  sync/1 -> chan_ctr/1
  din/1 -> Mux/2
  y_beam_val/1 -> real/1
  Convert/1 -> real/2
  zero_pad/1 -> real/3
  tv/1 -> Mux/3
  zero_pad1/1 -> imag/2
  imag/1 -> tv/2

== system x64_32s_001_4ks/beam_tvg/pow_sim_mux8 ==
  sync : Inport  OutDataType=fixdt(1, 16)  OutScaling=2^0
  din : Inport  Port=2  OutDataType=fixdt(1, 16)  OutScaling=2^0
  tvg_en : Inport  Port=3  OutDataType=fixdt(1, 16)  OutScaling=2^0
  new_window : Inport  Port=4  OutDataType=fixdt(1, 16)  OutScaling=2^0
  Convert : xbsIndex_r4/Convert  arith_type=Unsigned  n_bits=8  bin_pt=0  quantization=Truncate  overflow=Wrap  latency=0  dbl_ovrd=off  pipeline=off  block_type=convert
      settings: block_version=11.4
  Mux : xbsIndex_r4/Mux  inputs=2  en=off  latency=1  precision=Full  arith_type=Unsigned  n_bits=16  bin_pt=14  quantization=Truncate  overflow=Wrap  dbl_ovrd=off  block_type=mux
      settings: block_version=11.4
  beam_ctr : xbsIndex_r4/Counter  cnt_type=Free Running  cnt_to=Inf  operation=Up  start_count=0  cnt_by_val=1  arith_type=Unsigned  n_bits=ant_bits  bin_pt=0  load_pin=off  rst=on  en=off  explicit_period=on  period=1  dbl_ovrd=off  use_behavioral_HDL=off  implementation=Fabric  block_type=counter
      settings: block_version=11.4
  chan_ctr : xbsIndex_r4/Counter  cnt_type=Free Running  cnt_to=Inf  operation=Up  start_count=0  cnt_by_val=1  arith_type=Unsigned  n_bits=16  bin_pt=0  load_pin=off  rst=on  en=on  explicit_period=on  period=1  dbl_ovrd=off  use_behavioral_HDL=off  implementation=Fabric  block_type=counter
      settings: block_version=11.4
  imag : xbsIndex_r4/Concat  num_inputs=2  dbl_ovrd=off  block_type=concat
  real : xbsIndex_r4/Concat  num_inputs=3  dbl_ovrd=off  block_type=concat
  tv : xbsIndex_r4/Concat  num_inputs=2  dbl_ovrd=off  block_type=concat
  y_beam_val : xbsIndex_r4/Constant  arith_type=Unsigned  const=y_beam_val  n_bits=8  bin_pt=0  explicit_period=on  period=1  equ=P=C  opselect=C  inp2=PCIN>>17  opr=+  inp1=P  carry=CIN  dbl_ovrd=off  block_type=constant
      settings: block_version=11.4
  zero_pad : xbsIndex_r4/Constant  arith_type=Unsigned  const=0  n_bits=2  bin_pt=0  explicit_period=on  period=1  equ=P=C  opselect=C  inp2=PCIN>>17  opr=+  inp1=P  carry=CIN  dbl_ovrd=off  block_type=constant
      settings: block_version=11.4
  zero_pad1 : xbsIndex_r4/Constant  arith_type=Unsigned  const=0  n_bits=2  bin_pt=0  explicit_period=on  period=1  equ=P=C  opselect=C  inp2=PCIN>>17  opr=+  inp1=P  carry=CIN  dbl_ovrd=off  block_type=constant
      settings: block_version=11.4
  dout : Outport
  wires:
  imag/1 -> tv/2
  zero_pad1/1 -> imag/2
  tv/1 -> Mux/3
  zero_pad/1 -> real/3
  Convert/1 -> real/2
  y_beam_val/1 -> real/1
  din/1 -> Mux/2
  sync/1 -> chan_ctr/1
  beam_ctr/1 -> Convert/1
  tvg_en/1 -> Mux/1
  Mux/1 -> dout/1
  chan_ctr/1 -> imag/1
  real/1 -> tv/1
  new_window/1 -> chan_ctr/2, beam_ctr/1

== system x64_32s_001_4ks/beam_tvg/pow_sim_mux9 ==
  sync : Inport  OutDataType=fixdt(1, 16)  OutScaling=2^0
  din : Inport  Port=2  OutDataType=fixdt(1, 16)  OutScaling=2^0
  tvg_en : Inport  Port=3  OutDataType=fixdt(1, 16)  OutScaling=2^0
  new_window : Inport  Port=4  OutDataType=fixdt(1, 16)  OutScaling=2^0
  Convert : xbsIndex_r4/Convert  arith_type=Unsigned  n_bits=8  bin_pt=0  quantization=Truncate  overflow=Wrap  latency=0  dbl_ovrd=off  pipeline=off  block_type=convert
      settings: block_version=11.4
  Mux : xbsIndex_r4/Mux  inputs=2  en=off  latency=1  precision=Full  arith_type=Unsigned  n_bits=16  bin_pt=14  quantization=Truncate  overflow=Wrap  dbl_ovrd=off  block_type=mux
      settings: block_version=11.4
  beam_ctr : xbsIndex_r4/Counter  cnt_type=Free Running  cnt_to=Inf  operation=Up  start_count=0  cnt_by_val=1  arith_type=Unsigned  n_bits=ant_bits  bin_pt=0  load_pin=off  rst=on  en=off  explicit_period=on  period=1  dbl_ovrd=off  use_behavioral_HDL=off  implementation=Fabric  block_type=counter
      settings: block_version=11.4
  chan_ctr : xbsIndex_r4/Counter  cnt_type=Free Running  cnt_to=Inf  operation=Up  start_count=0  cnt_by_val=1  arith_type=Unsigned  n_bits=16  bin_pt=0  load_pin=off  rst=on  en=on  explicit_period=on  period=1  dbl_ovrd=off  use_behavioral_HDL=off  implementation=Fabric  block_type=counter
      settings: block_version=11.4
  imag : xbsIndex_r4/Concat  num_inputs=2  dbl_ovrd=off  block_type=concat
  real : xbsIndex_r4/Concat  num_inputs=3  dbl_ovrd=off  block_type=concat
  tv : xbsIndex_r4/Concat  num_inputs=2  dbl_ovrd=off  block_type=concat
  y_beam_val : xbsIndex_r4/Constant  arith_type=Unsigned  const=y_beam_val  n_bits=8  bin_pt=0  explicit_period=on  period=1  equ=P=C  opselect=C  inp2=PCIN>>17  opr=+  inp1=P  carry=CIN  dbl_ovrd=off  block_type=constant
      settings: block_version=11.4
  zero_pad : xbsIndex_r4/Constant  arith_type=Unsigned  const=0  n_bits=2  bin_pt=0  explicit_period=on  period=1  equ=P=C  opselect=C  inp2=PCIN>>17  opr=+  inp1=P  carry=CIN  dbl_ovrd=off  block_type=constant
      settings: block_version=11.4
  zero_pad1 : xbsIndex_r4/Constant  arith_type=Unsigned  const=0  n_bits=2  bin_pt=0  explicit_period=on  period=1  equ=P=C  opselect=C  inp2=PCIN>>17  opr=+  inp1=P  carry=CIN  dbl_ovrd=off  block_type=constant
      settings: block_version=11.4
  dout : Outport
  wires:
  new_window/1 -> beam_ctr/1, chan_ctr/2
  real/1 -> tv/1
  chan_ctr/1 -> imag/1
  Mux/1 -> dout/1
  tvg_en/1 -> Mux/1
  beam_ctr/1 -> Convert/1
  sync/1 -> chan_ctr/1
  din/1 -> Mux/2
  y_beam_val/1 -> real/1
  Convert/1 -> real/2
  zero_pad/1 -> real/3
  tv/1 -> Mux/3
  zero_pad1/1 -> imag/2
  imag/1 -> tv/2
